simulink model "large_model"  (Simulink 8.0) (continued)
== system large_model/Integrated_GPM2_4/GPM == (continued)
  ads_4A/3 -> [ADS4A_3_ads_4A]  "<ads_4A>"
  [ADS4A_3_ads_4A] -> Self_checking_pair_ADS4A/3(In3)
  ads_4A/2 -> [ADS4A_2_ads_4A]  "<ads_4A>"
  [ADS4A_2_ads_4A] -> Self_checking_pair_ADS4A/2(In2)
  ads_4A/1 -> [ADS4A_1_ads_4A]  "<ads_4A>"
  [ADS4A_1_ads_4A] -> Self_checking_pair_ADS4A/1(In1)
  ads_3B/8 -> [ADS3B_8_ads_3B]  "<ads_3B>"
  [ADS3B_8_ads_3B] -> Self_checking_pair_ADS3B/8(In8)
  ads_3B/7 -> [ADS3B_7_ads_3B]  "<ads_3B>"
  [ADS3B_7_ads_3B] -> Self_checking_pair_ADS3B/7(In7)
  ads_3B/6 -> [ADS3B_6_ads_3B]  "<ads_3B>"
  [ADS3B_6_ads_3B] -> Self_checking_pair_ADS3B/6(In6)
  ads_3B/5 -> [ADS3B_5_ads_3B]  "<ads_3B>"
  [ADS3B_5_ads_3B] -> Self_checking_pair_ADS3B/5(In5)
  ads_3B/4 -> [ADS3B_4_ads_3B]  "<ads_3B>"
  [ADS3B_4_ads_3B] -> Self_checking_pair_ADS3B/4(In4)
  ads_3B/3 -> [ADS3B_3_ads_3B]  "<ads_3B>"
  [ADS3B_3_ads_3B] -> Self_checking_pair_ADS3B/3(In3)
  ads_3B/2 -> [ADS3B_2_ads_3B]  "<ads_3B>"
  [ADS3B_2_ads_3B] -> Self_checking_pair_ADS3B/2(In2)
  ads_3B/1 -> [ADS3B_1_ads_3B]  "<ads_3B>"
  [ADS3B_1_ads_3B] -> Self_checking_pair_ADS3B/1(In1)
  ads_3A/8 -> [ADS3A_8_ads_3A]  "<ads_3A>"
  [ADS3A_8_ads_3A] -> Self_checking_pair_ADS3A/8(In8)
  ads_3A/7 -> [ADS3A_7_ads_3A]  "<ads_3A>"
  [ADS3A_7_ads_3A] -> Self_checking_pair_ADS3A/7(In7)
  ads_3A/6 -> [ADS3A_6_ads_3A]  "<ads_3A>"
  [ADS3A_6_ads_3A] -> Self_checking_pair_ADS3A/6(In6)
  ads_3A/5 -> [ADS3A_5_ads_3A]  "<ads_3A>"
  [ADS3A_5_ads_3A] -> Self_checking_pair_ADS3A/5(In5)
  ads_3A/4 -> [ADS3A_4_ads_3A]  "<ads_3A>"
  [ADS3A_4_ads_3A] -> Self_checking_pair_ADS3A/4(In4)
  ads_3A/3 -> [ADS3A_3_ads_3A]  "<ads_3A>"
  [ADS3A_3_ads_3A] -> Self_checking_pair_ADS3A/3(In3)
  ads_3A/2 -> [ADS3A_2_ads_3A]  "<ads_3A>"
  [ADS3A_2_ads_3A] -> Self_checking_pair_ADS3A/2(In2)
  ads_3A/1 -> [ADS3A_1_ads_3A]  "<ads_3A>"
  [ADS3A_1_ads_3A] -> Self_checking_pair_ADS3A/1(In1)
  ads_2B/8 -> [ADS2B_8_ads_2B]  "<ads_2B>"
  [ADS2B_8_ads_2B] -> Self_checking_pair_ADS2B/8(In8)
  ads_2B/7 -> [ADS2B_7_ads_2B]  "<ads_2B>"
  [ADS2B_7_ads_2B] -> Self_checking_pair_ADS2B/7(In7)
  ads_2B/6 -> [ADS2B_6_ads_2B]  "<ads_2B>"
  [ADS2B_6_ads_2B] -> Self_checking_pair_ADS2B/6(In6)
  ads_2B/5 -> [ADS2B_5_ads_2B]  "<ads_2B>"
  [ADS2B_5_ads_2B] -> Self_checking_pair_ADS2B/5(In5)
  ads_2B/4 -> [ADS2B_4_ads_2B]  "<ads_2B>"
  [ADS2B_4_ads_2B] -> Self_checking_pair_ADS2B/4(In4)
  ads_2B/3 -> [ADS2B_3_ads_2B]  "<ads_2B>"
  [ADS2B_3_ads_2B] -> Self_checking_pair_ADS2B/3(In3)
  ads_2B/2 -> [ADS2B_2_ads_2B]  "<ads_2B>"
  [ADS2B_2_ads_2B] -> Self_checking_pair_ADS2B/2(In2)
  ads_2B/1 -> [ADS2B_1_ads_2B]  "<ads_2B>"
  [ADS2B_1_ads_2B] -> Self_checking_pair_ADS2B/1(In1)
  ads_2A/8 -> [ADS2A_8_ads_2A]  "<ads_2A>"
  [ADS2A_8_ads_2A] -> Self_checking_pair_ADS2A/8(In8)
  ads_2A/7 -> [ADS2A_7_ads_2A]  "<ads_2A>"
  [ADS2A_7_ads_2A] -> Self_checking_pair_ADS2A/7(In7)
  ads_2A/6 -> [ADS2A_6_ads_2A]  "<ads_2A>"
  [ADS2A_6_ads_2A] -> Self_checking_pair_ADS2A/6(In6)
  ads_2A/5 -> [ADS2A_5_ads_2A]  "<ads_2A>"
  [ADS2A_5_ads_2A] -> Self_checking_pair_ADS2A/5(In5)
  ads_2A/4 -> [ADS2A_4_ads_2A]  "<ads_2A>"
  [ADS2A_4_ads_2A] -> Self_checking_pair_ADS2A/4(In4)
  ads_2A/3 -> [ADS2A_3_ads_2A]  "<ads_2A>"
  [ADS2A_3_ads_2A] -> Self_checking_pair_ADS2A/3(In3)
  ads_2A/2 -> [ADS2A_2_ads_2A]  "<ads_2A>"
  [ADS2A_2_ads_2A] -> Self_checking_pair_ADS2A/2(In2)
  ads_2A/1 -> [ADS2A_1_ads_2A]  "<ads_2A>"
  [ADS2A_1_ads_2A] -> Self_checking_pair_ADS2A/1(In1)
  ads_1B/8 -> [ADS1B_8_ads_1B]  "<ads_1B>"
  [ADS1B_8_ads_1B] -> Self_checking_pair_ADS1B/8(In8)
  ads_1B/7 -> [ADS1B_7_ads_1B]  "<ads_1B>"
  [ADS1B_7_ads_1B] -> Self_checking_pair_ADS1B/7(In7)
  ads_1B/6 -> [ADS1B_6_ads_1B]  "<ads_1B>"
  [ADS1B_6_ads_1B] -> Self_checking_pair_ADS1B/6(In6)
  ads_1B/5 -> [ADS1B_5_ads_1B]  "<ads_1B>"
  [ADS1B_5_ads_1B] -> Self_checking_pair_ADS1B/5(In5)
  ads_1B/4 -> [ADS1B_4_ads_1B]  "<ads_1B>"
  [ADS1B_4_ads_1B] -> Self_checking_pair_ADS1B/4(In4)
  ads_1B/3 -> [ADS1B_3_ads_1B]  "<ads_1B>"
  [ADS1B_3_ads_1B] -> Self_checking_pair_ADS1B/3(In3)
  ads_1B/2 -> [ADS1B_2_ads_1B]  "<ads_1B>"
  [ADS1B_2_ads_1B] -> Self_checking_pair_ADS1B/2(In2)
  ads_1B/1 -> [ADS1B_1_ads_1B]  "<ads_1B>"
  [ADS1B_1_ads_1B] -> Self_checking_pair_ADS1B/1(In1)
  ads_1A/8 -> [ADS1A_8_ads_1A]  "<ads_1A>"
  [ADS1A_8_ads_1A] -> Self_checking_pair_ADS1A/8(In8)
  ads_1A/7 -> [ADS1A_7_ads_1A]  "<ads_1A>"
  [ADS1A_7_ads_1A] -> Self_checking_pair_ADS1A/7(In7)
  ads_1A/6 -> [ADS1A_6_ads_1A]  "<ads_1A>"
  [ADS1A_6_ads_1A] -> Self_checking_pair_ADS1A/6(In6)
  ads_1A/5 -> [ADS1A_5_ads_1A]  "<ads_1A>"
  [ADS1A_5_ads_1A] -> Self_checking_pair_ADS1A/5(In5)
  ads_1A/4 -> [ADS1A_4_ads_1A]  "<ads_1A>"
  [ADS1A_4_ads_1A] -> Self_checking_pair_ADS1A/4(In4)
  ads_1A/3 -> [ADS1A_3_ads_1A]  "<ads_1A>"
  [ADS1A_3_ads_1A] -> Self_checking_pair_ADS1A/3(In3)
  ads_1A/2 -> [ADS1A_2_ads_1A]  "<ads_1A>"
  [ADS1A_2_ads_1A] -> Self_checking_pair_ADS1A/2(In2)
  ads_1A/1 -> [ADS1A_1_ads_1A]  "<ads_1A>"
  [ADS1A_1_ads_1A] -> Self_checking_pair_ADS1A/1(In1)
  sidestick2_pitch_1/8 -> [sidestick2_pitch_1_8_sidestick2_pitch_1]  "<sidestick2_pitch_1>"
  [sidestick2_pitch_1_8_sidestick2_pitch_1] -> Self_checking_pair_SSC2_pitch1/8(In8)
  sidestick2_pitch_1/7 -> [sidestick2_pitch_1_7_sidestick2_pitch_1]  "<sidestick2_pitch_1>"
  [sidestick2_pitch_1_7_sidestick2_pitch_1] -> Self_checking_pair_SSC2_pitch1/7(In7)
  sidestick2_pitch_1/6 -> [sidestick2_pitch_1_6_sidestick2_pitch_1]  "<sidestick2_pitch_1>"
  [sidestick2_pitch_1_6_sidestick2_pitch_1] -> Self_checking_pair_SSC2_pitch1/6(In6)
  sidestick2_pitch_1/5 -> [sidestick2_pitch_1_5_sidestick2_pitch_1]  "<sidestick2_pitch_1>"
  [sidestick2_pitch_1_5_sidestick2_pitch_1] -> Self_checking_pair_SSC2_pitch1/5(In5)
  sidestick2_pitch_1/4 -> [sidestick2_pitch_1_4_sidestick2_pitch_1]  "<sidestick2_pitch_1>"
  [sidestick2_pitch_1_4_sidestick2_pitch_1] -> Self_checking_pair_SSC2_pitch1/4(In4)
  sidestick2_pitch_1/3 -> [sidestick2_pitch_1_3_sidestick2_pitch_1]  "<sidestick2_pitch_1>"
  [sidestick2_pitch_1_3_sidestick2_pitch_1] -> Self_checking_pair_SSC2_pitch1/3(In3)
  sidestick2_pitch_1/2 -> [sidestick2_pitch_1_2_sidestick2_pitch_1]  "<sidestick2_pitch_1>"
  [sidestick2_pitch_1_2_sidestick2_pitch_1] -> Self_checking_pair_SSC2_pitch1/2(In2)
  sidestick2_pitch_1/1 -> [sidestick2_pitch_1_1_sidestick2_pitch_1]  "<sidestick2_pitch_1>"
  [sidestick2_pitch_1_1_sidestick2_pitch_1] -> Self_checking_pair_SSC2_pitch1/1(In1)
  sidestick1_pitch_4/8 -> [sidestick1_pitch_4_8_sidestick1_pitch_4]  "<sidestick1_pitch_4>"
  [sidestick1_pitch_4_8_sidestick1_pitch_4] -> Self_checking_pair_SSC1_pitch4/8(In8)
  sidestick1_pitch_4/7 -> [sidestick1_pitch_4_7_sidestick1_pitch_4]  "<sidestick1_pitch_4>"
  [sidestick1_pitch_4_7_sidestick1_pitch_4] -> Self_checking_pair_SSC1_pitch4/7(In7)
  sidestick1_pitch_4/6 -> [sidestick1_pitch_4_6_sidestick1_pitch_4]  "<sidestick1_pitch_4>"
  [sidestick1_pitch_4_6_sidestick1_pitch_4] -> Self_checking_pair_SSC1_pitch4/6(In6)
  sidestick1_pitch_4/5 -> [sidestick1_pitch_4_5_sidestick1_pitch_4]  "<sidestick1_pitch_4>"
  [sidestick1_pitch_4_5_sidestick1_pitch_4] -> Self_checking_pair_SSC1_pitch4/5(In5)
  sidestick1_pitch_4/4 -> [sidestick1_pitch_4_4_sidestick1_pitch_4]  "<sidestick1_pitch_4>"
  [sidestick1_pitch_4_4_sidestick1_pitch_4] -> Self_checking_pair_SSC1_pitch4/4(In4)
  sidestick1_pitch_4/3 -> [sidestick1_pitch_4_3_sidestick1_pitch_4]  "<sidestick1_pitch_4>"
  [sidestick1_pitch_4_3_sidestick1_pitch_4] -> Self_checking_pair_SSC1_pitch4/3(In3)
  sidestick1_pitch_4/2 -> [sidestick1_pitch_4_2_sidestick1_pitch_4]  "<sidestick1_pitch_4>"
  [sidestick1_pitch_4_2_sidestick1_pitch_4] -> Self_checking_pair_SSC1_pitch4/2(In2)
  sidestick1_pitch_4/1 -> [sidestick1_pitch_4_1_sidestick1_pitch_4]  "<sidestick1_pitch_4>"
  [sidestick1_pitch_4_1_sidestick1_pitch_4] -> Self_checking_pair_SSC1_pitch4/1(In1)
  sidestick1_pitch_3/8 -> [sidestick1_pitch_3_8_sidestick1_pitch_3]  "<sidestick1_pitch_3>"
  [sidestick1_pitch_3_8_sidestick1_pitch_3] -> Self_checking_pair_SSC1_pitch3/8(In8)
  sidestick1_pitch_3/7 -> [sidestick1_pitch_3_7_sidestick1_pitch_3]  "<sidestick1_pitch_3>"
  [sidestick1_pitch_3_7_sidestick1_pitch_3] -> Self_checking_pair_SSC1_pitch3/7(In7)
  sidestick1_pitch_3/6 -> [sidestick1_pitch_3_6_sidestick1_pitch_3]  "<sidestick1_pitch_3>"
  [sidestick1_pitch_3_6_sidestick1_pitch_3] -> Self_checking_pair_SSC1_pitch3/6(In6)
  sidestick1_pitch_3/5 -> [sidestick1_pitch_3_5_sidestick1_pitch_3]  "<sidestick1_pitch_3>"
  [sidestick1_pitch_3_5_sidestick1_pitch_3] -> Self_checking_pair_SSC1_pitch3/5(In5)
  sidestick1_pitch_3/4 -> [sidestick1_pitch_3_4_sidestick1_pitch_3]  "<sidestick1_pitch_3>"
  [sidestick1_pitch_3_4_sidestick1_pitch_3] -> Self_checking_pair_SSC1_pitch3/4(In4)
  sidestick1_pitch_3/3 -> [sidestick1_pitch_3_3_sidestick1_pitch_3]  "<sidestick1_pitch_3>"
  [sidestick1_pitch_3_3_sidestick1_pitch_3] -> Self_checking_pair_SSC1_pitch3/3(In3)
  sidestick1_pitch_3/2 -> [sidestick1_pitch_3_2_sidestick1_pitch_3]  "<sidestick1_pitch_3>"
  [sidestick1_pitch_3_2_sidestick1_pitch_3] -> Self_checking_pair_SSC1_pitch3/2(In2)
  sidestick1_pitch_3/1 -> [sidestick1_pitch_3_1_sidestick1_pitch_3]  "<sidestick1_pitch_3>"
  [sidestick1_pitch_3_1_sidestick1_pitch_3] -> Self_checking_pair_SSC1_pitch3/1(In1)
  sidestick2_roll_4/8 -> [sidestick2_roll_4_8_sidestick2_roll_4]  "<sidestick2_roll_4>"
  [sidestick2_roll_4_8_sidestick2_roll_4] -> Self_checking_pair_SSC2_roll4/8(In8)
  sidestick2_roll_4/7 -> [sidestick2_roll_4_7_sidestick2_roll_4]  "<sidestick2_roll_4>"
  [sidestick2_roll_4_7_sidestick2_roll_4] -> Self_checking_pair_SSC2_roll4/7(In7)
  sidestick2_roll_4/6 -> [sidestick2_roll_4_6_sidestick2_roll_4]  "<sidestick2_roll_4>"
  [sidestick2_roll_4_6_sidestick2_roll_4] -> Self_checking_pair_SSC2_roll4/6(In6)
  sidestick2_roll_4/5 -> [sidestick2_roll_4_5_sidestick2_roll_4]  "<sidestick2_roll_4>"
  [sidestick2_roll_4_5_sidestick2_roll_4] -> Self_checking_pair_SSC2_roll4/5(In5)
  sidestick2_roll_4/4 -> [sidestick2_roll_4_4_sidestick2_roll_4]  "<sidestick2_roll_4>"
  [sidestick2_roll_4_4_sidestick2_roll_4] -> Self_checking_pair_SSC2_roll4/4(In4)
  sidestick2_roll_4/3 -> [sidestick2_roll_4_3_sidestick2_roll_4]  "<sidestick2_roll_4>"
  [sidestick2_roll_4_3_sidestick2_roll_4] -> Self_checking_pair_SSC2_roll4/3(In3)
  sidestick2_roll_4/2 -> [sidestick2_roll_4_2_sidestick2_roll_4]  "<sidestick2_roll_4>"
  [sidestick2_roll_4_2_sidestick2_roll_4] -> Self_checking_pair_SSC2_roll4/2(In2)
  sidestick2_roll_4/1 -> [sidestick2_roll_4_1_sidestick2_roll_4]  "<sidestick2_roll_4>"
  [sidestick2_roll_4_1_sidestick2_roll_4] -> Self_checking_pair_SSC2_roll4/1(In1)
  sidestick2_roll_3/8 -> [sidestick2_roll_3_8_sidestick2_roll_3]  "<sidestick2_roll_3>"
  [sidestick2_roll_3_8_sidestick2_roll_3] -> Self_checking_pair_SSC2_roll3/8(In8)
  sidestick2_roll_3/7 -> [sidestick2_roll_3_7_sidestick2_roll_3]  "<sidestick2_roll_3>"
  [sidestick2_roll_3_7_sidestick2_roll_3] -> Self_checking_pair_SSC2_roll3/7(In7)
  sidestick2_roll_3/6 -> [sidestick2_roll_3_6_sidestick2_roll_3]  "<sidestick2_roll_3>"
  [sidestick2_roll_3_6_sidestick2_roll_3] -> Self_checking_pair_SSC2_roll3/6(In6)
  sidestick2_roll_3/5 -> [sidestick2_roll_3_5_sidestick2_roll_3]  "<sidestick2_roll_3>"
  [sidestick2_roll_3_5_sidestick2_roll_3] -> Self_checking_pair_SSC2_roll3/5(In5)
  sidestick2_roll_3/4 -> [sidestick2_roll_3_4_sidestick2_roll_3]  "<sidestick2_roll_3>"
  [sidestick2_roll_3_4_sidestick2_roll_3] -> Self_checking_pair_SSC2_roll3/4(In4)
  sidestick2_roll_3/3 -> [sidestick2_roll_3_3_sidestick2_roll_3]  "<sidestick2_roll_3>"
  [sidestick2_roll_3_3_sidestick2_roll_3] -> Self_checking_pair_SSC2_roll3/3(In3)
  sidestick2_roll_3/2 -> [sidestick2_roll_3_2_sidestick2_roll_3]  "<sidestick2_roll_3>"
  [sidestick2_roll_3_2_sidestick2_roll_3] -> Self_checking_pair_SSC2_roll3/2(In2)
  sidestick2_roll_3/1 -> [sidestick2_roll_3_1_sidestick2_roll_3]  "<sidestick2_roll_3>"
  [sidestick2_roll_3_1_sidestick2_roll_3] -> Self_checking_pair_SSC2_roll3/1(In1)
  sidestick2_roll_2/8 -> [sidestick2_roll_2_8_sidestick2_roll_2]  "<sidestick2_roll_2>"
  [sidestick2_roll_2_8_sidestick2_roll_2] -> Self_checking_pair_SSC2_roll2/8(In8)
  sidestick2_roll_2/7 -> [sidestick2_roll_2_7_sidestick2_roll_2]  "<sidestick2_roll_2>"
  [sidestick2_roll_2_7_sidestick2_roll_2] -> Self_checking_pair_SSC2_roll2/7(In7)
  sidestick2_roll_2/6 -> [sidestick2_roll_2_6_sidestick2_roll_2]  "<sidestick2_roll_2>"
  [sidestick2_roll_2_6_sidestick2_roll_2] -> Self_checking_pair_SSC2_roll2/6(In6)
  sidestick2_roll_2/5 -> [sidestick2_roll_2_5_sidestick2_roll_2]  "<sidestick2_roll_2>"
  [sidestick2_roll_2_5_sidestick2_roll_2] -> Self_checking_pair_SSC2_roll2/5(In5)
  sidestick2_roll_2/4 -> [sidestick2_roll_2_4_sidestick2_roll_2]  "<sidestick2_roll_2>"
  [sidestick2_roll_2_4_sidestick2_roll_2] -> Self_checking_pair_SSC2_roll2/4(In4)
  sidestick2_roll_2/3 -> [sidestick2_roll_2_3_sidestick2_roll_2]  "<sidestick2_roll_2>"
  [sidestick2_roll_2_3_sidestick2_roll_2] -> Self_checking_pair_SSC2_roll2/3(In3)
  sidestick2_roll_2/2 -> [sidestick2_roll_2_2_sidestick2_roll_2]  "<sidestick2_roll_2>"
  [sidestick2_roll_2_2_sidestick2_roll_2] -> Self_checking_pair_SSC2_roll2/2(In2)
  sidestick2_roll_2/1 -> [sidestick2_roll_2_1_sidestick2_roll_2]  "<sidestick2_roll_2>"
  [sidestick2_roll_2_1_sidestick2_roll_2] -> Self_checking_pair_SSC2_roll2/1(In1)
  sidestick2_roll_1/8 -> [sidestick2_roll_1_8_sidestick2_roll_1]  "<sidestick2_roll_1>"
  [sidestick2_roll_1_8_sidestick2_roll_1] -> Self_checking_pair_SSC2_roll1/8(In8)
  sidestick2_roll_1/7 -> [sidestick2_roll_1_7_sidestick2_roll_1]  "<sidestick2_roll_1>"
  [sidestick2_roll_1_7_sidestick2_roll_1] -> Self_checking_pair_SSC2_roll1/7(In7)
  sidestick2_roll_1/6 -> [sidestick2_roll_1_6_sidestick2_roll_1]  "<sidestick2_roll_1>"
  [sidestick2_roll_1_6_sidestick2_roll_1] -> Self_checking_pair_SSC2_roll1/6(In6)
  sidestick2_roll_1/5 -> [sidestick2_roll_1_5_sidestick2_roll_1]  "<sidestick2_roll_1>"
  [sidestick2_roll_1_5_sidestick2_roll_1] -> Self_checking_pair_SSC2_roll1/5(In5)
  sidestick2_roll_1/4 -> [sidestick2_roll_1_4_sidestick2_roll_1]  "<sidestick2_roll_1>"
  [sidestick2_roll_1_4_sidestick2_roll_1] -> Self_checking_pair_SSC2_roll1/4(In4)
  sidestick2_roll_1/3 -> [sidestick2_roll_1_3_sidestick2_roll_1]  "<sidestick2_roll_1>"
  [sidestick2_roll_1_3_sidestick2_roll_1] -> Self_checking_pair_SSC2_roll1/3(In3)
  sidestick2_roll_1/2 -> [sidestick2_roll_1_2_sidestick2_roll_1]  "<sidestick2_roll_1>"
  [sidestick2_roll_1_2_sidestick2_roll_1] -> Self_checking_pair_SSC2_roll1/2(In2)
  sidestick2_roll_1/1 -> [sidestick2_roll_1_1_sidestick2_roll_1]  "<sidestick2_roll_1>"
  [sidestick2_roll_1_1_sidestick2_roll_1] -> Self_checking_pair_SSC2_roll1/1(In1)
  sidestick1_roll_4/8 -> [sidestick1_roll_4_8_sidestick1_roll_4]  "<sidestick1_roll_4>"
  [sidestick1_roll_4_8_sidestick1_roll_4] -> Self_checking_pair_SSC1_roll4/8(In8)
  sidestick1_roll_4/7 -> [sidestick1_roll_4_7_sidestick1_roll_4]  "<sidestick1_roll_4>"
  [sidestick1_roll_4_7_sidestick1_roll_4] -> Self_checking_pair_SSC1_roll4/7(In7)
  sidestick1_roll_4/6 -> [sidestick1_roll_4_6_sidestick1_roll_4]  "<sidestick1_roll_4>"
  [sidestick1_roll_4_6_sidestick1_roll_4] -> Self_checking_pair_SSC1_roll4/6(In6)
  sidestick1_roll_4/5 -> [sidestick1_roll_4_5_sidestick1_roll_4]  "<sidestick1_roll_4>"
  [sidestick1_roll_4_5_sidestick1_roll_4] -> Self_checking_pair_SSC1_roll4/5(In5)
  sidestick1_roll_4/4 -> [sidestick1_roll_4_4_sidestick1_roll_4]  "<sidestick1_roll_4>"
  [sidestick1_roll_4_4_sidestick1_roll_4] -> Self_checking_pair_SSC1_roll4/4(In4)
  sidestick1_roll_4/3 -> [sidestick1_roll_4_3_sidestick1_roll_4]  "<sidestick1_roll_4>"
  [sidestick1_roll_4_3_sidestick1_roll_4] -> Self_checking_pair_SSC1_roll4/3(In3)
  sidestick1_roll_4/2 -> [sidestick1_roll_4_2_sidestick1_roll_4]  "<sidestick1_roll_4>"
  [sidestick1_roll_4_2_sidestick1_roll_4] -> Self_checking_pair_SSC1_roll4/2(In2)
  sidestick1_roll_4/1 -> [sidestick1_roll_4_1_sidestick1_roll_4]  "<sidestick1_roll_4>"
  [sidestick1_roll_4_1_sidestick1_roll_4] -> Self_checking_pair_SSC1_roll4/1(In1)
  sidestick1_roll_3/8 -> [sidestick1_roll_3_8_sidestick1_roll_3]  "<sidestick1_roll_3>"
  [sidestick1_roll_3_8_sidestick1_roll_3] -> Self_checking_pair_SSC1_roll3/8(In8)
  sidestick1_roll_3/7 -> [sidestick1_roll_3_7_sidestick1_roll_3]  "<sidestick1_roll_3>"
  [sidestick1_roll_3_7_sidestick1_roll_3] -> Self_checking_pair_SSC1_roll3/7(In7)
  sidestick1_roll_3/6 -> [sidestick1_roll_3_6_sidestick1_roll_3]  "<sidestick1_roll_3>"
  [sidestick1_roll_3_6_sidestick1_roll_3] -> Self_checking_pair_SSC1_roll3/6(In6)
  sidestick1_roll_3/5 -> [sidestick1_roll_3_5_sidestick1_roll_3]  "<sidestick1_roll_3>"
  [sidestick1_roll_3_5_sidestick1_roll_3] -> Self_checking_pair_SSC1_roll3/5(In5)
  sidestick1_roll_3/4 -> [sidestick1_roll_3_4_sidestick1_roll_3]  "<sidestick1_roll_3>"
  [sidestick1_roll_3_4_sidestick1_roll_3] -> Self_checking_pair_SSC1_roll3/4(In4)
  sidestick1_roll_3/3 -> [sidestick1_roll_3_3_sidestick1_roll_3]  "<sidestick1_roll_3>"
  [sidestick1_roll_3_3_sidestick1_roll_3] -> Self_checking_pair_SSC1_roll3/3(In3)
  sidestick1_roll_3/2 -> [sidestick1_roll_3_2_sidestick1_roll_3]  "<sidestick1_roll_3>"
  [sidestick1_roll_3_2_sidestick1_roll_3] -> Self_checking_pair_SSC1_roll3/2(In2)
  sidestick1_roll_3/1 -> [sidestick1_roll_3_1_sidestick1_roll_3]  "<sidestick1_roll_3>"
  [sidestick1_roll_3_1_sidestick1_roll_3] -> Self_checking_pair_SSC1_roll3/1(In1)
  sidestick1_roll_2/8 -> [sidestick1_roll_2_8_sidestick1_roll_2]  "<sidestick1_roll_2>"
  [sidestick1_roll_2_8_sidestick1_roll_2] -> Self_checking_pair_SSC1_roll2/8(In8)
  sidestick1_roll_2/7 -> [sidestick1_roll_2_7_sidestick1_roll_2]  "<sidestick1_roll_2>"
  [sidestick1_roll_2_7_sidestick1_roll_2] -> Self_checking_pair_SSC1_roll2/7(In7)
  sidestick1_roll_2/6 -> [sidestick1_roll_2_6_sidestick1_roll_2]  "<sidestick1_roll_2>"
  [sidestick1_roll_2_6_sidestick1_roll_2] -> Self_checking_pair_SSC1_roll2/6(In6)
  sidestick1_roll_2/5 -> [sidestick1_roll_2_5_sidestick1_roll_2]  "<sidestick1_roll_2>"
  [sidestick1_roll_2_5_sidestick1_roll_2] -> Self_checking_pair_SSC1_roll2/5(In5)
  sidestick1_roll_2/4 -> [sidestick1_roll_2_4_sidestick1_roll_2]  "<sidestick1_roll_2>"
  [sidestick1_roll_2_4_sidestick1_roll_2] -> Self_checking_pair_SSC1_roll2/4(In4)
  sidestick1_roll_2/3 -> [sidestick1_roll_2_3_sidestick1_roll_2]  "<sidestick1_roll_2>"
  [sidestick1_roll_2_3_sidestick1_roll_2] -> Self_checking_pair_SSC1_roll2/3(In3)
  sidestick1_roll_2/2 -> [sidestick1_roll_2_2_sidestick1_roll_2]  "<sidestick1_roll_2>"
  [sidestick1_roll_2_2_sidestick1_roll_2] -> Self_checking_pair_SSC1_roll2/2(In2)
  sidestick1_roll_2/1 -> [sidestick1_roll_2_1_sidestick1_roll_2]  "<sidestick1_roll_2>"
  [sidestick1_roll_2_1_sidestick1_roll_2] -> Self_checking_pair_SSC1_roll2/1(In1)
  sidestick1_roll_1/4 -> [sidestick1_roll_1_4_sidestick1_roll_1]  "<sidestick1_roll_1>"
  [sidestick1_roll_1_4_sidestick1_roll_1] -> Self_checking_pair_SSC1_roll1/4(In4)
  sidestick1_roll_1/3 -> [sidestick1_roll_1_3_sidestick1_roll_1]  "<sidestick1_roll_1>"
  [sidestick1_roll_1_3_sidestick1_roll_1] -> Self_checking_pair_SSC1_roll1/3(In3)
  sidestick1_roll_1/2 -> [sidestick1_roll_1_2_sidestick1_roll_1]  "<sidestick1_roll_1>"
  [sidestick1_roll_1_2_sidestick1_roll_1] -> Self_checking_pair_SSC1_roll1/2(In2)
  sidestick1_roll_1/1 -> [sidestick1_roll_1_1_sidestick1_roll_1]  "<sidestick1_roll_1>"
  [sidestick1_roll_1_1_sidestick1_roll_1] -> Self_checking_pair_SSC1_roll1/1(In1)
  sidestick2_pitch_4/8 -> [sidestick2_pitch_4_8_sidestick2_pitch_4]  "<sidestick2_pitch_4>"
  [sidestick2_pitch_4_8_sidestick2_pitch_4] -> Self_checking_pair_SSC2_pitch4/8(In8)
  sidestick2_pitch_4/7 -> [sidestick2_pitch_4_7_sidestick2_pitch_4]  "<sidestick2_pitch_4>"
  [sidestick2_pitch_4_7_sidestick2_pitch_4] -> Self_checking_pair_SSC2_pitch4/7(In7)
  sidestick2_pitch_4/6 -> [sidestick2_pitch_4_6_sidestick2_pitch_4]  "<sidestick2_pitch_4>"
  [sidestick2_pitch_4_6_sidestick2_pitch_4] -> Self_checking_pair_SSC2_pitch4/6(In6)
  sidestick2_pitch_4/5 -> [sidestick2_pitch_4_5_sidestick2_pitch_4]  "<sidestick2_pitch_4>"
  [sidestick2_pitch_4_5_sidestick2_pitch_4] -> Self_checking_pair_SSC2_pitch4/5(In5)
  sidestick2_pitch_4/4 -> [sidestick2_pitch_4_4_sidestick2_pitch_4]  "<sidestick2_pitch_4>"
  [sidestick2_pitch_4_4_sidestick2_pitch_4] -> Self_checking_pair_SSC2_pitch4/4(In4)
  sidestick2_pitch_4/3 -> [sidestick2_pitch_4_3_sidestick2_pitch_4]  "<sidestick2_pitch_4>"
  [sidestick2_pitch_4_3_sidestick2_pitch_4] -> Self_checking_pair_SSC2_pitch4/3(In3)
  sidestick2_pitch_4/2 -> [sidestick2_pitch_4_2_sidestick2_pitch_4]  "<sidestick2_pitch_4>"
  [sidestick2_pitch_4_2_sidestick2_pitch_4] -> Self_checking_pair_SSC2_pitch4/2(In2)
  sidestick2_pitch_4/1 -> [sidestick2_pitch_4_1_sidestick2_pitch_4]  "<sidestick2_pitch_4>"
  [sidestick2_pitch_4_1_sidestick2_pitch_4] -> Self_checking_pair_SSC2_pitch4/1(In1)
  sidestick2_pitch_3/8 -> [sidestick2_pitch_3_8_sidestick2_pitch_3]  "<sidestick2_pitch_3>"
  [sidestick2_pitch_3_8_sidestick2_pitch_3] -> Self_checking_pair_SSC2_pitch3/8(In8)
  sidestick2_pitch_3/7 -> [sidestick2_pitch_3_7_sidestick2_pitch_3]  "<sidestick2_pitch_3>"
  [sidestick2_pitch_3_7_sidestick2_pitch_3] -> Self_checking_pair_SSC2_pitch3/7(In7)
  sidestick2_pitch_3/6 -> [sidestick2_pitch_3_6_sidestick2_pitch_3]  "<sidestick2_pitch_3>"
  [sidestick2_pitch_3_6_sidestick2_pitch_3] -> Self_checking_pair_SSC2_pitch3/6(In6)
  sidestick2_pitch_3/5 -> [sidestick2_pitch_3_5_sidestick2_pitch_3]  "<sidestick2_pitch_3>"
  [sidestick2_pitch_3_5_sidestick2_pitch_3] -> Self_checking_pair_SSC2_pitch3/5(In5)
  sidestick2_pitch_3/4 -> [sidestick2_pitch_3_4_sidestick2_pitch_3]  "<sidestick2_pitch_3>"
  [sidestick2_pitch_3_4_sidestick2_pitch_3] -> Self_checking_pair_SSC2_pitch3/4(In4)
  sidestick2_pitch_3/3 -> [sidestick2_pitch_3_3_sidestick2_pitch_3]  "<sidestick2_pitch_3>"
  [sidestick2_pitch_3_3_sidestick2_pitch_3] -> Self_checking_pair_SSC2_pitch3/3(In3)
  sidestick2_pitch_3/2 -> [sidestick2_pitch_3_2_sidestick2_pitch_3]  "<sidestick2_pitch_3>"
  [sidestick2_pitch_3_2_sidestick2_pitch_3] -> Self_checking_pair_SSC2_pitch3/2(In2)
  sidestick2_pitch_3/1 -> [sidestick2_pitch_3_1_sidestick2_pitch_3]  "<sidestick2_pitch_3>"
  [sidestick2_pitch_3_1_sidestick2_pitch_3] -> Self_checking_pair_SSC2_pitch3/1(In1)
  sidestick2_pitch_2/8 -> [sidestick2_pitch_2_8_sidestick2_pitch_2]  "<sidestick2_pitch_2>"
  [sidestick2_pitch_2_8_sidestick2_pitch_2] -> Self_checking_pair_SSC2_pitch2/8(In8)
  sidestick2_pitch_2/7 -> [sidestick2_pitch_2_7_sidestick2_pitch_2]  "<sidestick2_pitch_2>"
  [sidestick2_pitch_2_7_sidestick2_pitch_2] -> Self_checking_pair_SSC2_pitch2/7(In7)
  sidestick2_pitch_2/6 -> [sidestick2_pitch_2_6_sidestick2_pitch_2]  "<sidestick2_pitch_2>"
  [sidestick2_pitch_2_6_sidestick2_pitch_2] -> Self_checking_pair_SSC2_pitch2/6(In6)
  sidestick2_pitch_2/5 -> [sidestick2_pitch_2_5_sidestick2_pitch_2]  "<sidestick2_pitch_2>"
  [sidestick2_pitch_2_5_sidestick2_pitch_2] -> Self_checking_pair_SSC2_pitch2/5(In5)
  sidestick2_pitch_2/4 -> [sidestick2_pitch_2_4_sidestick2_pitch_2]  "<sidestick2_pitch_2>"
  [sidestick2_pitch_2_4_sidestick2_pitch_2] -> Self_checking_pair_SSC2_pitch2/4(In4)
  sidestick2_pitch_2/3 -> [sidestick2_pitch_2_3_sidestick2_pitch_2]  "<sidestick2_pitch_2>"
  [sidestick2_pitch_2_3_sidestick2_pitch_2] -> Self_checking_pair_SSC2_pitch2/3(In3)
  sidestick2_pitch_2/2 -> [sidestick2_pitch_2_2_sidestick2_pitch_2]  "<sidestick2_pitch_2>"
  [sidestick2_pitch_2_2_sidestick2_pitch_2] -> Self_checking_pair_SSC2_pitch2/2(In2)
  sidestick2_pitch_2/1 -> [sidestick2_pitch_2_1_sidestick2_pitch_2]  "<sidestick2_pitch_2>"
  [sidestick2_pitch_2_1_sidestick2_pitch_2] -> Self_checking_pair_SSC2_pitch2/1(In1)
  sidestick1_pitch_2/8 -> [sidestick1_pitch_2_8_sidestick1_pitch_2]  "<sidestick1_pitch_2>"
  [sidestick1_pitch_2_8_sidestick1_pitch_2] -> Self_checking_pair_SSC1_pitch2/8(In8)
  sidestick1_pitch_2/7 -> [sidestick1_pitch_2_7_sidestick1_pitch_2]  "<sidestick1_pitch_2>"
  [sidestick1_pitch_2_7_sidestick1_pitch_2] -> Self_checking_pair_SSC1_pitch2/7(In7)
  sidestick1_pitch_2/6 -> [sidestick1_pitch_2_6_sidestick1_pitch_2]  "<sidestick1_pitch_2>"
  [sidestick1_pitch_2_6_sidestick1_pitch_2] -> Self_checking_pair_SSC1_pitch2/6(In6)
  sidestick1_pitch_2/5 -> [sidestick1_pitch_2_5_sidestick1_pitch_2]  "<sidestick1_pitch_2>"
  [sidestick1_pitch_2_5_sidestick1_pitch_2] -> Self_checking_pair_SSC1_pitch2/5(In5)
  sidestick1_pitch_2/4 -> [sidestick1_pitch_2_4_sidestick1_pitch_2]  "<sidestick1_pitch_2>"
  [sidestick1_pitch_2_4_sidestick1_pitch_2] -> Self_checking_pair_SSC1_pitch2/4(In4)
  sidestick1_pitch_2/3 -> [sidestick1_pitch_2_3_sidestick1_pitch_2]  "<sidestick1_pitch_2>"
  [sidestick1_pitch_2_3_sidestick1_pitch_2] -> Self_checking_pair_SSC1_pitch2/3(In3)
  sidestick1_pitch_2/2 -> [sidestick1_pitch_2_2_sidestick1_pitch_2]  "<sidestick1_pitch_2>"
  [sidestick1_pitch_2_2_sidestick1_pitch_2] -> Self_checking_pair_SSC1_pitch2/2(In2)
  sidestick1_pitch_2/1 -> [sidestick1_pitch_2_1_sidestick1_pitch_2]  "<sidestick1_pitch_2>"
  [sidestick1_pitch_2_1_sidestick1_pitch_2] -> Self_checking_pair_SSC1_pitch2/1(In1)
  sidestick1_pitch_1/8 -> [sidestick1_pitch_1_8_sidestick1_pitch_1]  "<sidestick1_pitch_1>"
  [sidestick1_pitch_1_8_sidestick1_pitch_1] -> Self_checking_pair_SSC1_pitch1/8(In8)
  sidestick1_pitch_1/7 -> [sidestick1_pitch_1_7_sidestick1_pitch_1]  "<sidestick1_pitch_1>"
  [sidestick1_pitch_1_7_sidestick1_pitch_1] -> Self_checking_pair_SSC1_pitch1/7(In7)
  sidestick1_pitch_1/6 -> [sidestick1_pitch_1_6_sidestick1_pitch_1]  "<sidestick1_pitch_1>"
  [sidestick1_pitch_1_6_sidestick1_pitch_1] -> Self_checking_pair_SSC1_pitch1/6(In6)
  sidestick1_pitch_1/5 -> [sidestick1_pitch_1_5_sidestick1_pitch_1]  "<sidestick1_pitch_1>"
  [sidestick1_pitch_1_5_sidestick1_pitch_1] -> Self_checking_pair_SSC1_pitch1/5(In5)
  sidestick1_pitch_1/4 -> [sidestick1_pitch_1_4_sidestick1_pitch_1]  "<sidestick1_pitch_1>"
  [sidestick1_pitch_1_4_sidestick1_pitch_1] -> Self_checking_pair_SSC1_pitch1/4(In4)
  sidestick1_pitch_1/3 -> [sidestick1_pitch_1_3_sidestick1_pitch_1]  "<sidestick1_pitch_1>"
  [sidestick1_pitch_1_3_sidestick1_pitch_1] -> Self_checking_pair_SSC1_pitch1/3(In3)
  sidestick1_pitch_1/2 -> [sidestick1_pitch_1_2_sidestick1_pitch_1]  "<sidestick1_pitch_1>"
  [sidestick1_pitch_1_2_sidestick1_pitch_1] -> Self_checking_pair_SSC1_pitch1/2(In2)
  sidestick1_pitch_1/1 -> [sidestick1_pitch_1_1_sidestick1_pitch_1]  "<sidestick1_pitch_1>"
  [sidestick1_pitch_1_1_sidestick1_pitch_1] -> Self_checking_pair_SSC1_pitch1/1(In1)
  Self_checking_pair_SSC1_pitch1/1(Out) -> [sidestick1Pitch1_cmd]
  Self_checking_pair_SSC1_pitch2/1(Out) -> [sidestick1Pitch2_cmd]
  Self_checking_pair_SSC1_pitch3/1(Out) -> [sidestick1Pitch3_cmd]
  Self_checking_pair_SSC1_pitch4/1(Out) -> [sidestick1Pitch4_cmd]
  Self_checking_pair_SSC2_pitch1/1(Out) -> [sidestick2Pitch1_cmd]
  Self_checking_pair_SSC2_pitch2/1(Out) -> [sidestick2Pitch2_cmd]
  Self_checking_pair_SSC2_pitch3/1(Out) -> [sidestick2Pitch3_cmd]
  Self_checking_pair_SSC2_pitch4/1(Out) -> [sidestick2Pitch4_cmd]
  Self_checking_pair_SSC1_roll1/1(Out) -> [sidestick1Roll1_cmd]
  sidestick1_roll_1/8 -> [sidestick1_roll_1_8_sidestick1_roll_1]  "<sidestick1_roll_1>"
  [sidestick1_roll_1_8_sidestick1_roll_1] -> Self_checking_pair_SSC1_roll1/8(In8)
  sidestick1_roll_1/7 -> [sidestick1_roll_1_7_sidestick1_roll_1]  "<sidestick1_roll_1>"
  [sidestick1_roll_1_7_sidestick1_roll_1] -> Self_checking_pair_SSC1_roll1/7(In7)
  sidestick1_roll_1/6 -> [sidestick1_roll_1_6_sidestick1_roll_1]  "<sidestick1_roll_1>"
  [sidestick1_roll_1_6_sidestick1_roll_1] -> Self_checking_pair_SSC1_roll1/6(In6)
  sidestick1_roll_1/5 -> [sidestick1_roll_1_5_sidestick1_roll_1]  "<sidestick1_roll_1>"
  [sidestick1_roll_1_5_sidestick1_roll_1] -> Self_checking_pair_SSC1_roll1/5(In5)
  Self_checking_pair_SSC1_roll2/1(Out) -> [sidestick1Roll2_cmd]
  Self_checking_pair_SSC1_roll3/1(Out) -> [sidestick1Roll3_cmd]
  Self_checking_pair_SSC1_roll4/1(Out) -> [sidestick1Roll4_cmd]
  Self_checking_pair_SSC2_roll1/1(Out) -> [sidestick2Roll1_cmd]
  Self_checking_pair_SSC2_roll2/1(Out) -> [sidestick2Roll2_cmd]
  Self_checking_pair_SSC2_roll3/1(Out) -> [sidestick2Roll3_cmd]
  Self_checking_pair_SSC2_roll4/1(Out) -> [sidestick2Roll4_cmd]
  pedal_yaw_4/8 -> [pedal_yaw_4_8_pedal_yaw_4]  "<pedal_yaw_4>"
  [pedal_yaw_4_8_pedal_yaw_4] -> Self_checking_pair_PedalYaw4/8(In8)
  pedal_yaw_4/7 -> [pedal_yaw_4_7_pedal_yaw_4]  "<pedal_yaw_4>"
  [pedal_yaw_4_7_pedal_yaw_4] -> Self_checking_pair_PedalYaw4/7(In7)
  pedal_yaw_4/6 -> [pedal_yaw_4_6_pedal_yaw_4]  "<pedal_yaw_4>"
  [pedal_yaw_4_6_pedal_yaw_4] -> Self_checking_pair_PedalYaw4/6(In6)
  pedal_yaw_4/5 -> [pedal_yaw_4_5_pedal_yaw_4]  "<pedal_yaw_4>"
  [pedal_yaw_4_5_pedal_yaw_4] -> Self_checking_pair_PedalYaw4/5(In5)
  pedal_yaw_4/4 -> [pedal_yaw_4_4_pedal_yaw_4]  "<pedal_yaw_4>"
  [pedal_yaw_4_4_pedal_yaw_4] -> Self_checking_pair_PedalYaw4/4(In4)
  pedal_yaw_4/3 -> [pedal_yaw_4_3_pedal_yaw_4]  "<pedal_yaw_4>"
  [pedal_yaw_4_3_pedal_yaw_4] -> Self_checking_pair_PedalYaw4/3(In3)
  pedal_yaw_4/2 -> [pedal_yaw_4_2_pedal_yaw_4]  "<pedal_yaw_4>"
  [pedal_yaw_4_2_pedal_yaw_4] -> Self_checking_pair_PedalYaw4/2(In2)
  pedal_yaw_4/1 -> [pedal_yaw_4_1_pedal_yaw_4]  "<pedal_yaw_4>"
  [pedal_yaw_4_1_pedal_yaw_4] -> Self_checking_pair_PedalYaw4/1(In1)
  pedal_yaw_3/8 -> [pedal_yaw_3_8_pedal_yaw_3]  "<pedal_yaw_3>"
  [pedal_yaw_3_8_pedal_yaw_3] -> Self_checking_pair_PedalYaw3/8(In8)
  pedal_yaw_3/7 -> [pedal_yaw_3_7_pedal_yaw_3]  "<pedal_yaw_3>"
  [pedal_yaw_3_7_pedal_yaw_3] -> Self_checking_pair_PedalYaw3/7(In7)
  pedal_yaw_3/6 -> [pedal_yaw_3_6_pedal_yaw_3]  "<pedal_yaw_3>"
  [pedal_yaw_3_6_pedal_yaw_3] -> Self_checking_pair_PedalYaw3/6(In6)
  pedal_yaw_3/5 -> [pedal_yaw_3_5_pedal_yaw_3]  "<pedal_yaw_3>"
  [pedal_yaw_3_5_pedal_yaw_3] -> Self_checking_pair_PedalYaw3/5(In5)
  pedal_yaw_3/4 -> [pedal_yaw_3_4_pedal_yaw_3]  "<pedal_yaw_3>"
  [pedal_yaw_3_4_pedal_yaw_3] -> Self_checking_pair_PedalYaw3/4(In4)
  pedal_yaw_3/3 -> [pedal_yaw_3_3_pedal_yaw_3]  "<pedal_yaw_3>"
  [pedal_yaw_3_3_pedal_yaw_3] -> Self_checking_pair_PedalYaw3/3(In3)
  pedal_yaw_3/2 -> [pedal_yaw_3_2_pedal_yaw_3]  "<pedal_yaw_3>"
  [pedal_yaw_3_2_pedal_yaw_3] -> Self_checking_pair_PedalYaw3/2(In2)
  pedal_yaw_3/1 -> [pedal_yaw_3_1_pedal_yaw_3]  "<pedal_yaw_3>"
  [pedal_yaw_3_1_pedal_yaw_3] -> Self_checking_pair_PedalYaw3/1(In1)
  pedal_yaw_2/8 -> [pedal_yaw_2_8_pedal_yaw_2]  "<pedal_yaw_2>"
  [pedal_yaw_2_8_pedal_yaw_2] -> Self_checking_pair_PedalYaw2/8(In8)
  pedal_yaw_2/7 -> [pedal_yaw_2_7_pedal_yaw_2]  "<pedal_yaw_2>"
  [pedal_yaw_2_7_pedal_yaw_2] -> Self_checking_pair_PedalYaw2/7(In7)
  pedal_yaw_2/6 -> [pedal_yaw_2_6_pedal_yaw_2]  "<pedal_yaw_2>"
  [pedal_yaw_2_6_pedal_yaw_2] -> Self_checking_pair_PedalYaw2/6(In6)
  pedal_yaw_2/5 -> [pedal_yaw_2_5_pedal_yaw_2]  "<pedal_yaw_2>"
  [pedal_yaw_2_5_pedal_yaw_2] -> Self_checking_pair_PedalYaw2/5(In5)
  pedal_yaw_2/4 -> [pedal_yaw_2_4_pedal_yaw_2]  "<pedal_yaw_2>"
  [pedal_yaw_2_4_pedal_yaw_2] -> Self_checking_pair_PedalYaw2/4(In4)
  pedal_yaw_2/3 -> [pedal_yaw_2_3_pedal_yaw_2]  "<pedal_yaw_2>"
  [pedal_yaw_2_3_pedal_yaw_2] -> Self_checking_pair_PedalYaw2/3(In3)
  pedal_yaw_2/2 -> [pedal_yaw_2_2_pedal_yaw_2]  "<pedal_yaw_2>"
  [pedal_yaw_2_2_pedal_yaw_2] -> Self_checking_pair_PedalYaw2/2(In2)
  pedal_yaw_2/1 -> [pedal_yaw_2_1_pedal_yaw_2]  "<pedal_yaw_2>"
  [pedal_yaw_2_1_pedal_yaw_2] -> Self_checking_pair_PedalYaw2/1(In1)
  pedal_yaw_1/8 -> [pedal_yaw_1_8_pedal_yaw_1]  "<pedal_yaw_1>"
  [pedal_yaw_1_8_pedal_yaw_1] -> Self_checking_pair_PedalYaw1/8(In8)
  pedal_yaw_1/7 -> [pedal_yaw_1_7_pedal_yaw_1]  "<pedal_yaw_1>"
  [pedal_yaw_1_7_pedal_yaw_1] -> Self_checking_pair_PedalYaw1/7(In7)
  pedal_yaw_1/6 -> [pedal_yaw_1_6_pedal_yaw_1]  "<pedal_yaw_1>"
  [pedal_yaw_1_6_pedal_yaw_1] -> Self_checking_pair_PedalYaw1/6(In6)
  pedal_yaw_1/5 -> [pedal_yaw_1_5_pedal_yaw_1]  "<pedal_yaw_1>"
  [pedal_yaw_1_5_pedal_yaw_1] -> Self_checking_pair_PedalYaw1/5(In5)
  pedal_yaw_1/4 -> [pedal_yaw_1_4_pedal_yaw_1]  "<pedal_yaw_1>"
  [pedal_yaw_1_4_pedal_yaw_1] -> Self_checking_pair_PedalYaw1/4(In4)
  pedal_yaw_1/3 -> [pedal_yaw_1_3_pedal_yaw_1]  "<pedal_yaw_1>"
  [pedal_yaw_1_3_pedal_yaw_1] -> Self_checking_pair_PedalYaw1/3(In3)
  pedal_yaw_1/2 -> [pedal_yaw_1_2_pedal_yaw_1]  "<pedal_yaw_1>"
  [pedal_yaw_1_2_pedal_yaw_1] -> Self_checking_pair_PedalYaw1/2(In2)
  pedal_yaw_1/1 -> [pedal_yaw_1_1_pedal_yaw_1]  "<pedal_yaw_1>"
  [pedal_yaw_1_1_pedal_yaw_1] -> Self_checking_pair_PedalYaw1/1(In1)
  ail_REU_LIB_LVDT_dat/1 -> SCP_ail_REU_LIB_LVDT/1(In1)  "<ail_REU_LIB_LVDT_dat>"
  ail_REU_LIB_LVDT_dat/2 -> SCP_ail_REU_LIB_LVDT/2(In2)  "<ail_REU_LIB_LVDT_dat>"
  ail_REU_LIB_mon_dat/1 -> SCP_ail_REU_LIB_mon/1(In1)  "<ail_REU_LIB_mon_dat>"
  ail_REU_LIB_mon_dat/2 -> SCP_ail_REU_LIB_mon/2(In2)  "<ail_REU_LIB_mon_dat>"
  ail_REU_LOB_LVDT_dat/1 -> SCP_ail_REU_LOB_LVDT/1(In1)  "<ail_REU_LOB_LVDT_dat>"
  ail_REU_LOB_LVDT_dat/2 -> SCP_ail_REU_LOB_LVDT/2(In2)  "<ail_REU_LOB_LVDT_dat>"
  ail_REU_LOB_mon_dat/1 -> SCP_ail_REU_LOB_mon/1(In1)  "<ail_REU_LOB_mon_dat>"
  ail_REU_LOB_mon_dat/2 -> SCP_ail_REU_LOB_mon/2(In2)  "<ail_REU_LOB_mon_dat>"
  ail_REU_RIB_LVDT_dat/1 -> SCP_ail_REU_RIB_LVDT/1(In1)  "<ail_REU_RIB_LVDT_dat>"
  ail_REU_RIB_LVDT_dat/2 -> SCP_ail_REU_RIB_LVDT/2(In2)  "<ail_REU_RIB_LVDT_dat>"
  ail_REU_RIB_mon_dat/1 -> SCP_ail_REU_RIB_mon/1(In1)  "<ail_REU_RIB_mon_dat>"
  ail_REU_RIB_mon_dat/2 -> SCP_ail_REU_RIB_mon/2(In2)  "<ail_REU_RIB_mon_dat>"
  ail_REU_ROB_LVDT_dat/1 -> SCP_REU_ROB_LVDT/1(In1)  "<ail_REU_ROB_LVDT_dat>"
  ail_REU_ROB_LVDT_dat/2 -> SCP_REU_ROB_LVDT/2(In2)  "<ail_REU_ROB_LVDT_dat>"
  ail_REU_ROB_mon_dat/1 -> SCP_ail_REU_ROB_mon/1(In1)  "<ail_REU_ROB_mon_dat>"
  ail_REU_ROB_mon_dat/2 -> SCP_ail_REU_ROB_mon/2(In2)  "<ail_REU_ROB_mon_dat>"
  elev_REU_LIB_LVDT_dat/1 -> SCP_elev_REU_LIB_LVDT/1(In1)  "<elev_REU_LIB_LVDT_dat>"
  elev_REU_LIB_LVDT_dat/2 -> SCP_elev_REU_LIB_LVDT/2(In2)  "<elev_REU_LIB_LVDT_dat>"
  elev_REU_LIB_mon_dat/1 -> SCP_elev_REU_LIB_mon_dat/1(In1)  "<elev_REU_LIB_mon_dat>"
  elev_REU_LIB_mon_dat/2 -> SCP_elev_REU_LIB_mon_dat/2(In2)  "<elev_REU_LIB_mon_dat>"
  elev_REU_LOB_LVDT_dat/1 -> SCP_elev_REU_LOB_LVDT/1(In1)  "<elev_REU_LOB_LVDT_dat>"
  elev_REU_LOB_LVDT_dat/2 -> SCP_elev_REU_LOB_LVDT/2(In2)  "<elev_REU_LOB_LVDT_dat>"
  elev_REU_LOB_mon_dat/1 -> SCP_elev_REU_LOB_mon/1(In1)  "<elev_REU_LOB_mon_dat>"
  elev_REU_LOB_mon_dat/2 -> SCP_elev_REU_LOB_mon/2(In2)  "<elev_REU_LOB_mon_dat>"
  rud_REU1_LVDT_dat/1 -> SCP_rud_REU1_LVDT/1(In1)  "<rud_REU1_LVDT_dat>"
  rud_REU1_LVDT_dat/2 -> SCP_rud_REU1_LVDT/2(In2)  "<rud_REU1_LVDT_dat>"
  rud_REU1_MON_dat/1 -> SCP_rud_REU1_MON/1(In1)  "<rud_REU1_MON_dat>"
  rud_REU1_MON_dat/2 -> SCP_rud_REU1_MON/2(In2)  "<rud_REU1_MON_dat>"
  rud_REU2_LVDT_dat/1 -> SCP_rud_REU2_LVDT/1(In1)  "<rud_REU2_LVDT_dat>"
  rud_REU2_LVDT_dat/2 -> SCP_rud_REU2_LVDT/2(In2)  "<rud_REU2_LVDT_dat>"
  rud_REU2_MON_dat/1 -> SCP_rud_REU2_MON/1(In1)  "<rud_REU2_MON_dat>"
  rud_REU2_MON_dat/2 -> SCP_rud_REU2_MON/2(In2)  "<rud_REU2_MON_dat>"
